simulink model "TosAeb"  (R2021a)
settings: Solver=FixedStepDiscrete  StartTime=0.0  StopTime=inf  FixedStep=0.01  RelTol=1e-3
undefined symbols (not defined in the file or in its folder): ACCModSts, AccSts1, AEB_ObjectMovingOrientation, AEB_ObjectMovingState, CN_BTCActive, CN_CurrentGearR, CN_CurrentGearR_DHT, CN_PTCActive, CN_VDCActive, CN_VSC_EspOff, CN_VSC_FCW_AEB_Error, CN_VSC_FCW_AEB_NoDispay, CN_VSC_FCW_AEB_PerfmDgdtn, CN_VSC_FCW_AEB_TempUnAvlbl, CN_VSC_VehStandstill, CNaccelAvoidMax, CNaccelAvoidMin, CNbrakePedalPress, CNcomfortStrLeftAccelMax, CNcomfortStrLeftAccelMin, CNcomfortStrRightAccelMax, CNcomfortStrRightAccelMin, CNDrvDoorOpen, CNDrvModeEco, CNDrvModeNorm, CNDrvModeOther, CNDrvModeSport, CNDrvSBRreminder, CNNull, CNobjInvalidID, CNPassDoorOpen, CNPassSBRreminder, CNPEBSAccelNegInf, CNPEBSAccelNull, CNPEBSTimeInf, CNPEBSTimeNull, CNSTOPMAX, CNSTOPNULL, CNsubjWidth, CNSwtOFF, CNSwtON, CNTTCMAX, CNTTCNULL, CNVehConfigAEBCycNone, CNVehConfigAEBNone, CNVehConfigAEBPedNone, CNVehConfigFCWNone, CNVehConfigJANone, CollisionSeverity, FALSE, FCWSnvty, JACycObjCalcInfoDefaultParams, JAPedObjCalcInfoDefaultParams, JAVehObjCalcInfoDefaultParams, latCycObjCalcInfoDefaultParams, latPedObjCalcInfoDefaultParams, longObjCalcInfoDefaultParams, ModSts, movingStatus, ObjectType ... (+1112)
libraries: simulink x3031, AEBSFunctionLib x50, Ta_Library x33, AEBSStateMachineLib x23, Mylibrary x12, simulink_extras x7, ObjSelect_lib x3, dspstat3 x2
(part 69 of 102)

== system TosAeb/FcwAebJa_Step_sys/ALG_AEBSystem/ALG_AEBController/AEB_JaObjCalcApp/JAVehTgtInfoCalc/AEBJAVehObjCalc/JAVehObjInfoCalc/ObjCrossFlgCal/CollisionProbCalc/Subsystem/ObjPreReferPointCalc ==
  dx : Inport  OutDataTypeStr=single
  vxAbsObj : Inport  Port=2  OutDataTypeStr=single
  tPredTime : Inport  Port=3  OutDataTypeStr=single
  axAbsObj : Inport  Port=4  OutDataTypeStr=single
  vy : Inport  Port=5  OutDataTypeStr=single
  subjLatAccel : Inport  Port=6
  ay : Inport  Port=7  OutDataTypeStr=single
  dy : Inport  Port=8  OutDataTypeStr=single
  ObjectYawAngle : Inport  Port=9  OutDataTypeStr=single
  Add : Sum  Inputs=+++  OutDataTypeStr=single
  Add1 : Sum  Inputs=+++  OutDataTypeStr=single
  Add2 : Sum  OutDataTypeStr=single
  Degrees to Radians : simulink_extras/Transformations/Degrees to Radians
  Gain : Gain  Gain=0.5  OutDataTypeStr=single
  Gain1 : Gain  Gain=0.5  OutDataTypeStr=single
  Math Function : Math  Operator=square  SignedPower=on  OutDataTypeStr=single  SaturateOnIntegerOverflow=on
  Math Function1 : Math  Operator=square  SignedPower=on  OutDataTypeStr=single  SaturateOnIntegerOverflow=on
  Product{,1..3} (4 alike) : Product  OutDataTypeStr=single
  dxPreRefPointObj : Outport
  dyPreRefPointObj : Outport  Port=2
  YawAnglePreObj : Outport  Port=3
  wires:
  vxAbsObj/1 -> Product/1
  tPredTime/1 -> Product/2, Math Function/1, Math Function1/1, Product2/2
  Product/1 -> Add/2
  Math Function/1 -> Product1/1
  axAbsObj/1 -> Product1/2
  Product1/1 -> Gain/1
  Gain/1 -> Add/3
  Add/1 -> dxPreRefPointObj/1
  dx/1 -> Add/1
  vy/1 -> Product2/1
  Math Function1/1 -> Product3/1
  Product3/1 -> Gain1/1
  Product2/1 -> Add1/1
  Gain1/1 -> Add1/2
  dy/1 -> Add1/3
  Add1/1 -> dyPreRefPointObj/1
  subjLatAccel/1 -> Add2/1
  ay/1 -> Add2/2
  Add2/1 -> Product3/2
  Degrees to Radians/1 -> YawAnglePreObj/1
  ObjectYawAngle/1 -> Degrees to Radians/1

== system TosAeb/FcwAebJa_Step_sys/ALG_AEBSystem/ALG_AEBController/AEB_JaObjCalcApp/JAVehTgtInfoCalc/AEBJAVehObjCalc/JAVehObjInfoCalc/ObjCrossFlgCal/CollisionProbCalc/Subsystem/PreCrossFlgCalcFlow/VectorCrossAlgorithm ==
  x1 : Inport  OutDataTypeStr=single
  y1 : Inport  Port=2  OutDataTypeStr=single
  x2 : Inport  Port=3  OutDataTypeStr=single
  y2 : Inport  Port=4  OutDataTypeStr=single
  m1 : Inport  Port=5  OutDataTypeStr=single
  n1 : Inport  Port=6  OutDataTypeStr=single
  m2 : Inport  Port=7  OutDataTypeStr=single
  n2 : Inport  Port=8  OutDataTypeStr=single
  f : TriggerPort  TriggerType=function-call  VariantControl=Variant
      settings: CopyFcn=Stateflow.SLINSF.SimulinkMan.removeBlockPreDeleteFcn  PreDeleteFcn=Stateflow.SLINSF.SimulinkMan.unsafePredeleteFcn
  Abs : Abs
  Bus Creator : BusCreator  Inputs=8
  Bus Selector : BusSelector  OutputSignals=x1,x2,n2,n1,y2,y1,m2,m1
  Compare To Constant : simulink/Logic and Bit Operations/Compare To Constant  relop=<  const=1e-6  OutDataTypeStr=boolean
  If : If  IfExpression=u1
  If Action Subsystem : Subsystem  TreatAsAtomicUnit=on
  If Action Subsystem1 : Subsystem  TreatAsAtomicUnit=on
  Merge : Merge
  VectorMulit : Subsystem
  preCrossFlg : Outport
  wires:
  x1/1 -> Bus Creator/1  "x1"
  y1/1 -> Bus Creator/2  "y1"
  x2/1 -> Bus Creator/3  "x2"
  y2/1 -> Bus Creator/4  "y2"
  m1/1 -> Bus Creator/5  "m1"
  n1/1 -> Bus Creator/6  "n1"
  m2/1 -> Bus Creator/7  "m2"
  n2/1 -> Bus Creator/8  "n2"
  Bus Creator/1 -> If Action Subsystem1/2(Coordinations), Bus Selector/1
  VectorMulit/1(vectorMulit) -> If Action Subsystem1/1(vectorMulit), Abs/1
  Abs/1 -> Compare To Constant/1
  Compare To Constant/1 -> If/1
  If/1 -> If Action Subsystem/Action
  If/2 -> If Action Subsystem1/Action
  If Action Subsystem/1(CrossFlg) -> Merge/1
  If Action Subsystem1/1(CrossFlg) -> Merge/2
  Merge/1 -> preCrossFlg/1
  Bus Selector/1 -> VectorMulit/1(x1)  "<x1>"
  Bus Selector/2 -> VectorMulit/2(x2)  "<x2>"
  Bus Selector/3 -> VectorMulit/3(n2)  "<n2>"
  Bus Selector/4 -> VectorMulit/4(n1)  "<n1>"
  Bus Selector/5 -> VectorMulit/5(y2)  "<y2>"
  Bus Selector/6 -> VectorMulit/6(y1)  "<y1>"
  Bus Selector/7 -> VectorMulit/7(m2)  "<m2>"
  Bus Selector/8 -> VectorMulit/8(m1)  "<m1>"

== system TosAeb/FcwAebJa_Step_sys/ALG_AEBSystem/ALG_AEBController/AEB_JaObjCalcApp/JAVehTgtInfoCalc/AEBJAVehObjCalc/JAVehObjInfoCalc/ObjCrossFlgCal/CollisionProbCalc/Subsystem/PreRefPointEgoCalc ==
  subjVel : Inport
  tPredTime : Inport  Port=2  OutDataTypeStr=single
  subjLongAccel : Inport  Port=3
  subjLatAccel : Inport  Port=4
  subjYawRate : Inport  Port=5
  Add : Sum  OutDataTypeStr=single
  Gain : Gain  Gain=0.5  OutDataTypeStr=single
  Gain1 : Gain  Gain=0.5  OutDataTypeStr=single
  Math Function : Math  Operator=square  SignedPower=on  OutDataTypeStr=single  SaturateOnIntegerOverflow=on
  Product{,1..3} (4 alike) : Product  OutDataTypeStr=single
  " dxPreRefPoint" : Outport
  " dyPreRefPoint" : Outport  Port=2
  YawAnglePre : Outport  Port=3
  wires:
  Gain1/1 -> " dyPreRefPoint"/1
  Gain/1 -> Add/2
  Product3/1 -> YawAnglePre/1
  subjYawRate/1 -> Product3/2
  Product2/1 -> Gain1/1
  subjLatAccel/1 -> Product2/2
  Add/1 -> " dxPreRefPoint"/1
  subjLongAccel/1 -> Product1/2
  Math Function/1 -> Product1/1, Product2/1
  tPredTime/1 -> Product3/1, Math Function/1, Product/2
  subjVel/1 -> Product/1
  Product/1 -> Add/1
  Product1/1 -> Gain/1

== system TosAeb/FcwAebJa_Step_sys/ALG_AEBSystem/ALG_AEBController/AEB_JaObjCalcApp/JAVehTgtInfoCalc/AEBJAVehObjCalc/JAVehObjInfoCalc/ObjCrossFlgCal/CollisionProbCalc/Subsystem/SubjBoundingBox ==
  dxPreRefPoint : Inport  OutDataTypeStr=single
  dyPreRefPoint : Inport  Port=2  OutDataTypeStr=single
  sincosCoef : Inport  Port=3
  Abs : Abs
  Bus Creator : BusCreator  Inputs=6
  Constant : Constant  Value=P_Global_DxvFrontBumper_m  OutDataTypeStr=single
  Constant1 : Constant  Value=P_Global_DxvRearBumper_m  OutDataTypeStr=single
  Constant2 : Constant  Value=P_Global_VehicleWidthMin_m  OutDataTypeStr=single
  Mux : Mux
  Mux1 : Mux
  SubjLLCoordinate : Subsystem
  SubjLUCoordinate : Subsystem
  SubjRLCoordinate : Subsystem
  SubjRUCoordinate : Subsystem
  subjDxPre : Outport
  subjDyPre : Outport  Port=2
  wires:
  Constant2/1 -> Bus Creator/6  "subjWidth"
  Abs/1 -> Bus Creator/5  "dxRearBumperToRearAxis"
  Constant/1 -> Bus Creator/4  "dxFrontBumperToRearAxis"
  sincosCoef/1 -> Bus Creator/3  "sincosCoef"
  dyPreRefPoint/1 -> Bus Creator/2  "dyPreRefPoint"
  dxPreRefPoint/1 -> Bus Creator/1  "dxPreRefPoint"
  Bus Creator/1 -> SubjRLCoordinate/1(inputSignals), SubjRUCoordinate/1(inputSignals), SubjLLCoordinate/1(inputSignals), SubjLUCoordinate/1(inputSignals)
  SubjLUCoordinate/1(dxPreLU) -> [dxPreLU]  "dxPreLU"
  SubjLUCoordinate/2(dyPreLU) -> [dyPreLU]  "dyPreLU"
  SubjLLCoordinate/1(dxPreLL) -> [dxPreLL]  "dxPreLL"
  SubjLLCoordinate/2(dyPreLL) -> [dyPreLL]  "dyPreLL"
  SubjRUCoordinate/2(dyPreRU) -> [dyPreRU]  "dyPreRU"
  SubjRLCoordinate/1(dxPreRL) -> [dxPreRL]  "dxPreRL"
  SubjRLCoordinate/2(dyPreRL) -> [dyPreRL]  "dyPreRL"
  Mux/1 -> subjDxPre/1
  Mux1/1 -> subjDyPre/1
  [dxPreRU] -> Mux/1
  [dxPreLU] -> Mux/2
  [dxPreLL] -> Mux/3
  [dxPreRL] -> Mux/4
  [dyPreRU] -> Mux1/1
  [dyPreLU] -> Mux1/2
  [dyPreRL] -> Mux1/4
  SubjRUCoordinate/1(dxPreRU) -> [dxPreRU]  "dyPreLU"
  [dyPreLL] -> Mux1/3
  Constant1/1 -> Abs/1

== system TosAeb/FcwAebJa_Step_sys/ALG_AEBSystem/ALG_AEBController/AEB_JaObjCalcApp/JAVehTgtInfoCalc/AEBJAVehObjCalc/JAVehObjInfoCalc/ObjCrossFlgCal/CollisionProbCalc/Subsystem/sincosCoefCalc ==
  angle : Inport  OutDataTypeStr=single
  Bus Creator : BusCreator  Inputs=2
  Sincos : Trigonometry  Operator=sincos
  sincosCoef : Outport
  wires:
  Sincos/1 -> Bus Creator/1  "sinCoef"
  angle/1 -> Sincos/1
  Sincos/2 -> Bus Creator/2  "cosCoef"
  Bus Creator/1 -> sincosCoef/1  "sincosCoef"

== system TosAeb/FcwAebJa_Step_sys/ALG_AEBSystem/ALG_AEBController/AEB_JaObjCalcApp/JAVehTgtInfoCalc/AEBJAVehObjCalc/JAVehObjInfoCalc/ObjCrossFlgCal/CollisionProbCalc/Subsystem/sincosCoefCalc1 ==
  angle : Inport  OutDataTypeStr=single
  Bus Creator : BusCreator  Inputs=2
  Sincos : Trigonometry  Operator=sincos
  sincosCoef : Outport
  wires:
  Bus Creator/1 -> sincosCoef/1  "sincosCoef"
  Sincos/2 -> Bus Creator/2  "cosCoef"
  angle/1 -> Sincos/1
  Sincos/1 -> Bus Creator/1  "sinCoef"

== system TosAeb/FcwAebJa_Step_sys/ALG_AEBSystem/ALG_AEBController/AEB_JaObjCalcApp/JAVehTgtInfoCalc/JAVehCollisonAssessInfoCalc/JaVehCollisonAssessInfoCalc/VehDistBrakeFullCalc/DistPhase3Calc/tPhase3Calc/tEgoStopCalc ==
  subjVel : Inport  OutDataTypeStr=single
  subjLongAccelFilt : Inport  Port=2  OutDataTypeStr=single
  tJerkWork : Inport  Port=3  OutDataTypeStr=single
  Kjerk : Inport  Port=4  OutDataTypeStr=single
  accelMax : Inport  Port=5  OutDataTypeStr=single
  Add : Sum  Inputs=3  OutDataTypeStr=single
  Constant : Constant  Value=0.5  OutDataTypeStr=single
  Divide : Product  Inputs=*/  OutDataTypeStr=single
  Gain : Gain  Gain=-1  OutDataTypeStr=single
  Product : Product  Inputs=4  OutDataTypeStr=single
  Product1 : Product  OutDataTypeStr=single
  tEgoStop : Outport
  wires:
  Divide/1 -> Gain/1
  Product/1 -> Add/3
  Product1/1 -> Add/2
  tJerkWork/1 -> Product/2, Product/1, Product1/2
  Add/1 -> Divide/1
  Gain/1 -> tEgoStop/1
  accelMax/1 -> Divide/2
  Kjerk/1 -> Product/3
  subjLongAccelFilt/1 -> Product1/1
  Constant/1 -> Product/4
  subjVel/1 -> Add/1

== system TosAeb/FcwAebJa_Step_sys/ALG_AEBSystem/ALG_AEBController/AEB_JaObjCalcApp/JAVehTgtInfoCalc/JAVehCollisonAssessInfoCalc/JaVehCollisonAssessInfoCalc/VehDistBrakeFullCalc/DistPhase3Calc/tPhase3Calc/tPhase3Calc ==
  tVelDelta : Inport  OutDataTypeStr=single
  tEgoStop : Inport  Port=2  OutDataTypeStr=single
  MinMax : MinMax  Inputs=2  OutDataTypeStr=single
  tPhase3 : Outport
  wires:
  tVelDelta/1 -> MinMax/1
  tEgoStop/1 -> MinMax/2
  MinMax/1 -> tPhase3/1

== system TosAeb/FcwAebJa_Step_sys/ALG_AEBSystem/ALG_AEBController/AEB_JaObjCalcApp/JAVehTgtInfoCalc/JAVehCollisonAssessInfoCalc/JaVehCollisonAssessInfoCalc/VehDistBrakeFullCalc/DistPhase3Calc/tPhase3Calc/tVelDeltaCalc ==
  velDelta : Inport  OutDataTypeStr=single
  subjLongAccelFilt : Inport  Port=2  OutDataTypeStr=single
  tJerkWork : Inport  Port=3  OutDataTypeStr=single
  Kjerk : Inport  Port=4  OutDataTypeStr=single
  accelMax : Inport  Port=5  OutDataTypeStr=single
  Add : Sum  Inputs=+--  OutDataTypeStr=single
  Constant : Constant  Value=0.5  OutDataTypeStr=single
  Divide : Product  Inputs=*/  OutDataTypeStr=single
  Product : Product  Inputs=4  OutDataTypeStr=single
  Product1 : Product  OutDataTypeStr=single
  tVelDelta : Outport
  wires:
  Divide/1 -> tVelDelta/1
  accelMax/1 -> Divide/2
  Kjerk/1 -> Product/3
  velDelta/1 -> Add/1
  Add/1 -> Divide/1
  Product1/1 -> Add/2
  Constant/1 -> Product/4
  tJerkWork/1 -> Product/2, Product/1, Product1/2
  Product/1 -> Add/3
  subjLongAccelFilt/1 -> Product1/1

== system TosAeb/FcwAebJa_Step_sys/ALG_AEBSystem/ALG_AEBController/AEB_JaObjCalcApp/JaCycTgtInfoCalc/JaCycCollisonAssessInfo/JaCycCollisonAssessInfoCalc/PedDistBrakeFullCalc/DistPhase3Calc/tPhase3Calc/tEgoStopCalc ==
  subjVel : Inport  OutDataTypeStr=single
  subjLongAccelFilt : Inport  Port=2  OutDataTypeStr=single
  tJerkWork : Inport  Port=3  OutDataTypeStr=single
  Kjerk : Inport  Port=4  OutDataTypeStr=single
  accelMax : Inport  Port=5  OutDataTypeStr=single
  Add : Sum  Inputs=3  OutDataTypeStr=single
  Constant : Constant  Value=0.5  OutDataTypeStr=single
  Divide : Product  Inputs=*/  OutDataTypeStr=single
  Gain : Gain  Gain=-1  OutDataTypeStr=single
  Product : Product  Inputs=4  OutDataTypeStr=single
  Product1 : Product  OutDataTypeStr=single
  tEgoStop : Outport
  wires:
  Divide/1 -> Gain/1
  Product/1 -> Add/3
  Product1/1 -> Add/2
  tJerkWork/1 -> Product/2, Product/1, Product1/2
  Add/1 -> Divide/1
  Gain/1 -> tEgoStop/1
  accelMax/1 -> Divide/2
  Kjerk/1 -> Product/3
  subjLongAccelFilt/1 -> Product1/1
  Constant/1 -> Product/4
  subjVel/1 -> Add/1

== system TosAeb/FcwAebJa_Step_sys/ALG_AEBSystem/ALG_AEBController/AEB_JaObjCalcApp/JaCycTgtInfoCalc/JaCycCollisonAssessInfo/JaCycCollisonAssessInfoCalc/PedDistBrakeFullCalc/DistPhase3Calc/tPhase3Calc/tPhase3Calc ==
  tVelDelta : Inport  OutDataTypeStr=single
  tEgoStop : Inport  Port=2  OutDataTypeStr=single
  MinMax : MinMax  Inputs=2  OutDataTypeStr=single
  tPhase3 : Outport
  wires:
  tVelDelta/1 -> MinMax/1
  tEgoStop/1 -> MinMax/2
  MinMax/1 -> tPhase3/1

== system TosAeb/FcwAebJa_Step_sys/ALG_AEBSystem/ALG_AEBController/AEB_JaObjCalcApp/JaCycTgtInfoCalc/JaCycCollisonAssessInfo/JaCycCollisonAssessInfoCalc/PedDistBrakeFullCalc/DistPhase3Calc/tPhase3Calc/tVelDeltaCalc ==
  velDelta : Inport  OutDataTypeStr=single
  subjLongAccelFilt : Inport  Port=2  OutDataTypeStr=single
  tJerkWork : Inport  Port=3  OutDataTypeStr=single
  Kjerk : Inport  Port=4  OutDataTypeStr=single
  accelMax : Inport  Port=5  OutDataTypeStr=single
  Add : Sum  Inputs=+--  OutDataTypeStr=single
  Constant : Constant  Value=0.5  OutDataTypeStr=single
  Divide : Product  Inputs=*/  OutDataTypeStr=single
  Product : Product  Inputs=4  OutDataTypeStr=single
  Product1 : Product  OutDataTypeStr=single
  tVelDelta : Outport
  wires:
  Divide/1 -> tVelDelta/1
  accelMax/1 -> Divide/2
  Kjerk/1 -> Product/3
  velDelta/1 -> Add/1
  Add/1 -> Divide/1
  Product1/1 -> Add/2
  Constant/1 -> Product/4
  tJerkWork/1 -> Product/2, Product/1, Product1/2
  Product/1 -> Add/3
  subjLongAccelFilt/1 -> Product1/1

== system TosAeb/FcwAebJa_Step_sys/ALG_AEBSystem/ALG_AEBController/AEB_JaObjCalcApp/JaCycTgtInfoCalc/JaCycObjCalcInfoCalc/JaCycObjInfoCalc/ObjCrossFlgCal/CollisionProbCalc/Subsystem/ObjBoundingBox ==
  ObjLengthCorr : Inport  OutDataTypeStr=single
  ObjWidthCorr : Inport  Port=2  OutDataTypeStr=single
  dxPreRefPointObj : Inport  Port=3  OutDataTypeStr=single
  dyPreRefPointObj : Inport  Port=4  OutDataTypeStr=single
  sincosCoef : Inport  Port=5
  Bus Creator : BusCreator  Inputs=5
  Mux : Mux
  Mux1 : Mux
  ObjLLCoordinate : Subsystem
  ObjLUCoordinate : Subsystem
  ObjRLCoordinate : Subsystem
  ObjRUCoordinate : Subsystem
  objDxPre : Outport
  objDyPre : Outport  Port=2
  wires:
  ObjLengthCorr/1 -> Bus Creator/1  "ObjLengthCorr"
  ObjWidthCorr/1 -> Bus Creator/2  "ObjWidthCorr"
  dxPreRefPointObj/1 -> Bus Creator/3  "dxPreRefPointObj"
  dyPreRefPointObj/1 -> Bus Creator/4  "dyPreRefPointObj"
  sincosCoef/1 -> Bus Creator/5  "sincosCoef"
  Bus Creator/1 -> ObjRLCoordinate/1(inputSignals), ObjRUCoordinate/1(inputSignals), ObjLLCoordinate/1(inputSignals), ObjLUCoordinate/1(inputSignals)
  ObjLUCoordinate/1(dxPreLUObj) -> [dxPreLUObj]  "dxPreLUObj"
  ObjLUCoordinate/2(dyPreLUobj) -> [dyPreLUObj]  "dyPreLUObj"
  ObjLLCoordinate/1(dxPre_LLObj) -> [dxPreLLObj]  "dxPreLLObj"
  ObjLLCoordinate/2(dyPreLLObj) -> [dyPreLLObj]  "dyPreLLObj"
  ObjRUCoordinate/1(dxPreRUObj) -> [dxPreRUObj]  "dxPreRUObj"
  ObjRUCoordinate/2(dyPreRUObj) -> [dyPreRUObj]  "dyPreRUObj"
  ObjRLCoordinate/1(dxPreRLObj) -> [dxPreRLObj]  "dxPreRLObj"
  ObjRLCoordinate/2(dyPreRLObj) -> [dyPreRLObj]  "dyPreRLObj"
  Mux/1 -> objDxPre/1
  Mux1/1 -> objDyPre/1
  [dxPreRUObj] -> Mux/1  "dxPreRUObj"
  [dxPreLUObj] -> Mux/2
  [dxPreLLObj] -> Mux/3
  [dxPreRLObj] -> Mux/4
  [dyPreRUObj] -> Mux1/1
  [dyPreLUObj] -> Mux1/2
  [dyPreLLObj] -> Mux1/3
  [dyPreRLObj] -> Mux1/4

== system TosAeb/FcwAebJa_Step_sys/ALG_AEBSystem/ALG_AEBController/AEB_JaObjCalcApp/JaCycTgtInfoCalc/JaCycObjCalcInfoCalc/JaCycObjInfoCalc/ObjCrossFlgCal/CollisionProbCalc/Subsystem/ObjLenthWidthCalc ==
  ObjectYawAngleCorr : Inport  OutDataTypeStr=single
  ObjectTypeCameraConfirmed : Inport  Port=2
  ObjectLength : Inport  Port=3  OutDataTypeStr=single
  ObjectWidth : Inport  Port=4  OutDataTypeStr=single
  ObjectMovingOrientation : Inport  Port=5
  ObjLengthWidthCalc : Stateflow chart  Commented=on  TreatAsAtomicUnit=on
  ObjLengthWidthCalc1 : Stateflow chart  TreatAsAtomicUnit=on
  P_LatPedCA_CollisionRiskProbLengthThres : Constant  Value=P_AEB_JaCycCA_PreCollisionCycLengthThres
  P_LatPedCA_CollisionRiskProbWidthThres : Constant  Value=P_AEB_JaCycCA_PreCollisionCycWidthThres
  Radians to Degrees : simulink_extras/Transformations/Radians to Degrees
  ObjLengthCorr : Outport
  ObjWidthCorr : Outport  Port=2
  wires:
  P_LatPedCA_CollisionRiskProbWidthThres/1 -> ObjLengthWidthCalc1/7(P_LatPedCA_CollisionRiskProbWidthThres)
  ObjectTypeCameraConfirmed/1 -> ObjLengthWidthCalc1/2(ObjectTypeCameraConfirmed)
  P_LatPedCA_CollisionRiskProbLengthThres/1 -> ObjLengthWidthCalc1/6(P_LatPedCA_CollisionRiskProbLengthThres)
  ObjLengthWidthCalc1/1(ObjectLength) -> ObjLengthCorr/1
  ObjLengthWidthCalc1/2(ObjectWidth) -> ObjWidthCorr/1
  ObjectMovingOrientation/1 -> ObjLengthWidthCalc1/5(ObjectMovingOrientation)
  ObjectYawAngleCorr/1 -> Radians to Degrees/1
  ObjectLength/1 -> ObjLengthWidthCalc1/3(ObjectLengthIn)
  ObjectWidth/1 -> ObjLengthWidthCalc1/4(ObjectWidthIn)
  Radians to Degrees/1 -> ObjLengthWidthCalc1/1(ObjectYawAngle)

== system TosAeb/FcwAebJa_Step_sys/ALG_AEBSystem/ALG_AEBController/AEB_JaObjCalcApp/JaCycTgtInfoCalc/JaCycObjCalcInfoCalc/JaCycObjInfoCalc/ObjCrossFlgCal/CollisionProbCalc/Subsystem/ObjPreReferPointCalc ==
  dx : Inport  OutDataTypeStr=single
  vxAbsObj : Inport  Port=2  OutDataTypeStr=single
  tPredTime : Inport  Port=3  OutDataTypeStr=single
  vyRelCorr : Inport  Port=4  OutDataTypeStr=single
  dy : Inport  Port=5  OutDataTypeStr=single
  ObjectYawObjectYawAngleCorrAngle : Inport  Port=6  OutDataTypeStr=single
  Add : Sum  OutDataTypeStr=single
  Add1 : Sum  OutDataTypeStr=single
  Product : Product  OutDataTypeStr=single
  Product2 : Product  OutDataTypeStr=single
  dxPreRefPointObj : Outport
  dyPreRefPointObj : Outport  Port=2
  YawAnglePreObj : Outport  Port=3
  wires:
  vxAbsObj/1 -> Product/1
  tPredTime/1 -> Product/2, Product2/2
  Product/1 -> Add/2
  Add/1 -> dxPreRefPointObj/1
  dx/1 -> Add/1
  vyRelCorr/1 -> Product2/1
  Product2/1 -> Add1/1
  dy/1 -> Add1/2
  Add1/1 -> dyPreRefPointObj/1
  ObjectYawObjectYawAngleCorrAngle/1 -> YawAnglePreObj/1

== system TosAeb/FcwAebJa_Step_sys/ALG_AEBSystem/ALG_AEBController/AEB_JaObjCalcApp/JaCycTgtInfoCalc/JaCycObjCalcInfoCalc/JaCycObjInfoCalc/ObjCrossFlgCal/CollisionProbCalc/Subsystem/PreCrossFlgCalcFlow/VectorCrossAlgorithm ==
  x1 : Inport  OutDataTypeStr=single
  y1 : Inport  Port=2  OutDataTypeStr=single
  x2 : Inport  Port=3  OutDataTypeStr=single
  y2 : Inport  Port=4  OutDataTypeStr=single
  m1 : Inport  Port=5  OutDataTypeStr=single
  n1 : Inport  Port=6  OutDataTypeStr=single
  m2 : Inport  Port=7  OutDataTypeStr=single
  n2 : Inport  Port=8  OutDataTypeStr=single
  f : TriggerPort  TriggerType=function-call  VariantControl=Variant
      settings: CopyFcn=Stateflow.SLINSF.SimulinkMan.removeBlockPreDeleteFcn  PreDeleteFcn=Stateflow.SLINSF.SimulinkMan.unsafePredeleteFcn
  Abs : Abs
  Bus Creator : BusCreator  Inputs=8
  Bus Selector : BusSelector  OutputSignals=x1,x2,n2,n1,y2,y1,m2,m1
  Compare To Constant : simulink/Logic and Bit Operations/Compare To Constant  relop=<  const=1e-6  OutDataTypeStr=boolean
  If : If  IfExpression=u1
  If Action Subsystem : Subsystem  TreatAsAtomicUnit=on
  If Action Subsystem1 : Subsystem  TreatAsAtomicUnit=on
  Merge : Merge
  VectorMulit : Subsystem
  preCrossFlg : Outport
  wires:
  x1/1 -> Bus Creator/1  "x1"
  y1/1 -> Bus Creator/2  "y1"
  x2/1 -> Bus Creator/3  "x2"
  y2/1 -> Bus Creator/4  "y2"
  m1/1 -> Bus Creator/5  "m1"
  n1/1 -> Bus Creator/6  "n1"
  m2/1 -> Bus Creator/7  "m2"
  n2/1 -> Bus Creator/8  "n2"
  Bus Creator/1 -> If Action Subsystem1/2(Coordinations), Bus Selector/1
  VectorMulit/1(vectorMulit) -> If Action Subsystem1/1(vectorMulit), Abs/1
  Abs/1 -> Compare To Constant/1
  Compare To Constant/1 -> If/1
  If/1 -> If Action Subsystem/Action
  If/2 -> If Action Subsystem1/Action
  If Action Subsystem/1(CrossFlg) -> Merge/1
  If Action Subsystem1/1(CrossFlg) -> Merge/2
  Merge/1 -> preCrossFlg/1
  Bus Selector/1 -> VectorMulit/1(x1)  "<x1>"
  Bus Selector/2 -> VectorMulit/2(x2)  "<x2>"
  Bus Selector/3 -> VectorMulit/3(n2)  "<n2>"
  Bus Selector/4 -> VectorMulit/4(n1)  "<n1>"
  Bus Selector/5 -> VectorMulit/5(y2)  "<y2>"
  Bus Selector/6 -> VectorMulit/6(y1)  "<y1>"
  Bus Selector/7 -> VectorMulit/7(m2)  "<m2>"
  Bus Selector/8 -> VectorMulit/8(m1)  "<m1>"

== system TosAeb/FcwAebJa_Step_sys/ALG_AEBSystem/ALG_AEBController/AEB_JaObjCalcApp/JaCycTgtInfoCalc/JaCycObjCalcInfoCalc/JaCycObjInfoCalc/ObjCrossFlgCal/CollisionProbCalc/Subsystem/PreRefPointEgoCalc ==
  subjVel : Inport  OutDataTypeStr=single
  tPredTime : Inport  Port=2  OutDataTypeStr=single
  subjLongAccel : Inport  Port=3  OutDataTypeStr=single
  subjLatAccel : Inport  Port=4  OutDataTypeStr=single
  subjYawRate : Inport  Port=5  OutDataTypeStr=single
  Add : Sum  OutDataTypeStr=single
  Gain : Gain  Gain=0.5  OutDataTypeStr=single
  Gain1 : Gain  Gain=0.5  OutDataTypeStr=single
  Math Function : Math  Operator=square  SignedPower=on  OutDataTypeStr=single  SaturateOnIntegerOverflow=on
  Product{,1..3} (4 alike) : Product  OutDataTypeStr=single
  " dxPreRefPoint" : Outport
  " dyPreRefPoint" : Outport  Port=2
  YawAnglePre : Outport  Port=3
  wires:
  Gain1/1 -> " dyPreRefPoint"/1
  Gain/1 -> Add/2
  Product3/1 -> YawAnglePre/1
  subjYawRate/1 -> Product3/2
  Product2/1 -> Gain1/1
  subjLatAccel/1 -> Product2/2
  Add/1 -> " dxPreRefPoint"/1
  subjLongAccel/1 -> Product1/2
  Math Function/1 -> Product1/1, Product2/1
  tPredTime/1 -> Product3/1, Math Function/1, Product/2
  subjVel/1 -> Product/1
  Product/1 -> Add/1
  Product1/1 -> Gain/1

== system TosAeb/FcwAebJa_Step_sys/ALG_AEBSystem/ALG_AEBController/AEB_JaObjCalcApp/JaCycTgtInfoCalc/JaCycObjCalcInfoCalc/JaCycObjInfoCalc/ObjCrossFlgCal/CollisionProbCalc/Subsystem/SubjBoundingBox ==
  dxPreRefPoint : Inport  OutDataTypeStr=single
  dyPreRefPoint : Inport  Port=2  OutDataTypeStr=single
  sincosCoef : Inport  Port=3
  Abs : Abs
  Bus Creator : BusCreator  Inputs=6
  Constant : Constant  Value=P_Global_DxvFrontBumper_m  OutDataTypeStr=single
  Constant1 : Constant  Value=P_Global_DxvRearBumper_m  OutDataTypeStr=single
  Constant2 : Constant  Value=P_Global_VehicleWidthMin_m  OutDataTypeStr=single
  Mux : Mux
  Mux1 : Mux
  SubjLLCoordinate : Subsystem
  SubjLUCoordinate : Subsystem
  SubjRLCoordinate : Subsystem
  SubjRUCoordinate : Subsystem
  subjDxPre : Outport
  subjDyPre : Outport  Port=2
  wires:
  Constant2/1 -> Bus Creator/6  "subjWidth"
  Abs/1 -> Bus Creator/5  "dxRearBumperToRearAxis"
  Constant/1 -> Bus Creator/4  "dxFrontBumperToRearAxis"
  sincosCoef/1 -> Bus Creator/3  "sincosCoef"
  dyPreRefPoint/1 -> Bus Creator/2  "dyPreRefPoint"
  dxPreRefPoint/1 -> Bus Creator/1  "dxPreRefPoint"
  Bus Creator/1 -> SubjRLCoordinate/1(inputSignals), SubjRUCoordinate/1(inputSignals), SubjLLCoordinate/1(inputSignals), SubjLUCoordinate/1(inputSignals)
  SubjLUCoordinate/1(dxPreLU) -> [dxPreLU]  "dxPreLU"
  SubjLUCoordinate/2(dyPreLU) -> [dyPreLU]  "dyPreLU"
  SubjLLCoordinate/1(dxPreLL) -> [dxPreLL]  "dxPreLL"
  SubjLLCoordinate/2(dyPreLL) -> [dyPreLL]  "dyPreLL"
  SubjRUCoordinate/1(dxPreRU) -> [dxPreRU]  "dxPreRU"
  SubjRUCoordinate/2(dyPreRU) -> [dyPreRU]  "dyPreRU"
  SubjRLCoordinate/1(dxPreRL) -> [dxPreRL]  "dxPreRL"
  SubjRLCoordinate/2(dyPreRL) -> [dyPreRL]  "dyPreRL"
  Mux/1 -> subjDxPre/1
  Mux1/1 -> subjDyPre/1
  [dxPreRU] -> Mux/1
  [dxPreLU] -> Mux/2
  [dxPreLL] -> Mux/3
  [dxPreRL] -> Mux/4
  [dyPreRU] -> Mux1/1
  [dyPreLU] -> Mux1/2
  [dyPreLL] -> Mux1/3
  [dyPreRL] -> Mux1/4
  Constant1/1 -> Abs/1

== system TosAeb/FcwAebJa_Step_sys/ALG_AEBSystem/ALG_AEBController/AEB_JaObjCalcApp/JaCycTgtInfoCalc/JaCycObjCalcInfoCalc/JaCycObjInfoCalc/ObjCrossFlgCal/CollisionProbCalc/Subsystem/sincosCoefCalc ==
  angle : Inport  OutDataTypeStr=single
  Bus Creator : BusCreator  Inputs=2
  Sincos : Trigonometry  Operator=sincos
  sincosCoef : Outport
  wires:
  Sincos/1 -> Bus Creator/1  "sinCoef"
  angle/1 -> Sincos/1
  Sincos/2 -> Bus Creator/2  "cosCoef"
  Bus Creator/1 -> sincosCoef/1  "sincosCoef"

== system TosAeb/FcwAebJa_Step_sys/ALG_AEBSystem/ALG_AEBController/AEB_JaObjCalcApp/JaCycTgtInfoCalc/JaCycObjCalcInfoCalc/JaCycObjInfoCalc/ObjCrossFlgCal/CollisionProbCalc/Subsystem/sincosCoefCalc1 ==
  angle : Inport  OutDataTypeStr=single
  Bus Creator : BusCreator  Inputs=2
  Sincos : Trigonometry  Operator=sincos
  sincosCoef : Outport
  wires:
  Bus Creator/1 -> sincosCoef/1  "sincosCoef"
  Sincos/2 -> Bus Creator/2  "cosCoef"
  angle/1 -> Sincos/1
  Sincos/1 -> Bus Creator/1  "sinCoef"

== system TosAeb/FcwAebJa_Step_sys/ALG_AEBSystem/ALG_AEBController/AEB_LatObjInfoCalcApp/LatCycObjCalc/LatCycCollisonAssessInfoCalc/LatCycCollisonAssessInfoCalc/DistBrkCondCalc/CycDistBrakeFullCalc/DistPhase2Calc/distPhase2Calc ==
  tPhase2 : Inport  OutDataTypeStr=single
  subjAccelFilt : Inport  Port=2  OutDataTypeStr=single
  Kjerk : Inport  Port=3  OutDataTypeStr=single
  subjVel : Inport  Port=4
  Add : Sum  Inputs=3  OutDataTypeStr=single
  Constant : Constant  Value=1/6  OutDataTypeStr=single
  Constant1 : Constant  Value=0.5  OutDataTypeStr=single
  Product : Product  Inputs=5  OutDataTypeStr=single
  Product1 : Product  Inputs=4  OutDataTypeStr=single
  Product2 : Product  OutDataTypeStr=single
  distPhase2 : Outport
  wires:
  tPhase2/1 -> Product1/3, Product1/4, Product2/2, Product/1, Product/2, Product/3
  Kjerk/1 -> Product/4
  Constant/1 -> Product/5
  subjAccelFilt/1 -> Product1/2
  Constant1/1 -> Product1/1
  subjVel/1 -> Product2/1
  Product2/1 -> Add/2
  Product/1 -> Add/3
  Product1/1 -> Add/1
  Add/1 -> distPhase2/1

== system TosAeb/FcwAebJa_Step_sys/ALG_AEBSystem/ALG_AEBController/AEB_LatObjInfoCalcApp/LatCycObjCalc/LatCycCollisonAssessInfoCalc/LatCycCollisonAssessInfoCalc/DistBrkCondCalc/CycDistBrakeFullCalc/DistPhase2Calc/tPhase2Calc ==
  note: t jerk_amax = Max[0 ，（ a m ax - a ego ） /K jerk ] ；
  note: t jerk_0 = （ -a ego /K jer k ） + sqrt （（ a ego /K jer k ） ²- 2* （ v ego / K jer k ））
  subjVel : Inport
  Kjerk : Inport  Port=2  OutDataTypeStr=single
  subjLongAccelFilt : Inport  Port=3  OutDataTypeStr=single
  accelMax : Inport  Port=4  OutDataTypeStr=single
  Add : Sum  Inputs=+-  OutDataTypeStr=single
  Add1 : Sum  Inputs=-+  OutDataTypeStr=single
  Add2 : Sum  Inputs=+-  OutDataTypeStr=single
  Constant : Constant  Value=0  OutDataTypeStr=single
  Divide : Product  Inputs=*/  OutDataTypeStr=single
  Divide1 : Product  Inputs=*/  OutDataTypeStr=single
  Divide2 : Product  Inputs=*/  OutDataTypeStr=single
  Gain : Gain  Gain=2  OutDataTypeStr=single
  Math Function : Math  Operator=square  SignedPower=on  OutDataTypeStr=single  SaturateOnIntegerOverflow=on
  MinMax : MinMax  Function=max  Inputs=2  OutDataTypeStr=single
  MinMax1 : MinMax  Inputs=2  OutDataTypeStr=single
  Relational Operator : RelationalOperator  Operator=<=  OutDataTypeStr=boolean  RndMeth=Simplest
  Sqrt : Sqrt  OutDataTypeStr=single  SaturateOnIntegerOverflow=on
  egoStopFlg : Outport
  tJerkWork : Outport  Port=2
  tPhase2 : Outport  Port=3
  wires:
  Add2/1 -> Divide2/1
  MinMax/1 -> tJerkWork/1, Relational Operator/2, MinMax1/1  "tJerkWork"
  subjLongAccelFilt/1 -> Add2/2, Divide/1
  Sqrt/1 -> Add1/2
  Kjerk/1 -> Divide2/2, Divide/2, Divide1/2
  accelMax/1 -> Add2/1
  Divide2/1 -> MinMax/1
  Constant/1 -> MinMax/2
  Divide/1 -> Add1/1, Math Function/1
  MinMax1/1 -> tPhase2/1
  Divide1/1 -> Gain/1
  Add/1 -> Sqrt/1
  Gain/1 -> Add/2
  Add1/1 -> Relational Operator/1, MinMax1/2  "tEgoStop"
  Math Function/1 -> Add/1
  subjVel/1 -> Divide1/1
  Relational Operator/1 -> egoStopFlg/1

== system TosAeb/FcwAebJa_Step_sys/ALG_AEBSystem/ALG_AEBController/AEB_LatObjInfoCalcApp/LatCycObjCalc/LatCycCollisonAssessInfoCalc/LatCycCollisonAssessInfoCalc/DistBrkCondCalc/CycDistBrakeFullCalc/DistPhase3Calc/distPhase3Calc ==
  note: d3=（vego+ 0.5* aego*（t1 + t2）+ 0.5* Kjerk* t2²）*t3 + 0.5* amax* t3²；
  tPhase3 : Inport  OutDataTypeStr=single
  subjVel : Inport  Port=2
  subjLongAccelFilt : Inport  Port=3  OutDataTypeStr=single
  Kjerk : Inport  Port=4  OutDataTypeStr=single
  tPhase1 : Inport  Port=5  OutDataTypeStr=single
  tPhase2 : Inport  Port=6  OutDataTypeStr=single
  accelMax : Inport  Port=7  OutDataTypeStr=single
  Add : Sum  OutDataTypeStr=single
  Add1 : Sum  Inputs=+++  OutDataTypeStr=single
  Add2 : Sum  OutDataTypeStr=single
  Constant : Constant  Value=0.5  OutDataTypeStr=single
  Constant1 : Constant  Value=0.5  OutDataTypeStr=single
  Constant2 : Constant  Value=0.5  OutDataTypeStr=single
  Product : Product  Inputs=3  OutDataTypeStr=single
  Product1 : Product  Inputs=4  OutDataTypeStr=single
  Product2 : Product  OutDataTypeStr=single
  Product3 : Product  Inputs=4  OutDataTypeStr=single
  distPhase3 : Outport
  wires:
  tPhase1/1 -> Add/1
  tPhase2/1 -> Product1/2, Product1/1, Add/2
  subjLongAccelFilt/1 -> Product/2
  Add/1 -> Product/3
  Constant/1 -> Product/1
  Constant1/1 -> Product1/4
  Kjerk/1 -> Product1/3
  Product/1 -> Add1/2
  Product1/1 -> Add1/3
  subjVel/1 -> Add1/1
  Add1/1 -> Product2/2
  tPhase3/1 -> Product2/1, Product3/4, Product3/3
  Constant2/1 -> Product3/1
  accelMax/1 -> Product3/2
  Product3/1 -> Add2/1
  Product2/1 -> Add2/2
  Add2/1 -> distPhase3/1

== system TosAeb/FcwAebJa_Step_sys/ALG_AEBSystem/ALG_AEBController/AEB_LatObjInfoCalcApp/LatCycObjCalc/LatCycCollisonAssessInfoCalc/LatCycCollisonAssessInfoCalc/DistBrkCondCalc/CycDistBrakeFullCalc/DistPhase3Calc/tPhase3Calc ==
  subjVel : Inport
  subjLongAccelFilt : Inport  Port=2  OutDataTypeStr=single
  tJerkWork : Inport  Port=3  OutDataTypeStr=single
  Kjerk : Inport  Port=4  OutDataTypeStr=single
  accelMax : Inport  Port=5  OutDataTypeStr=single
  velDelta : Inport  Port=6  OutDataTypeStr=single
  Action Port : ActionPort  ActionPortLabel=else
  Bus Creator : BusCreator  Inputs=6
  Bus Selector : BusSelector  OutputSignals=velDelta,subjLongAccelFilt,tJerkWork,Kjerk,accelMax
  Bus Selector1 : BusSelector  OutputSignals=subjVel,subjLongAccelFilt,tJerkWork,Kjerk,accelMax
  tEgoStopCalc : Subsystem
  tPhase3Calc : Subsystem
  tVelDeltaCalc : Subsystem
  tPhase3 : Outport
  wires:
  tVelDeltaCalc/1(tVelDelta) -> tPhase3Calc/1(tVelDelta)
  tEgoStopCalc/1(tEgoStop) -> tPhase3Calc/2(tEgoStop)
  tPhase3Calc/1(tPhase3) -> tPhase3/1
  subjVel/1 -> Bus Creator/1  "subjVel"
  subjLongAccelFilt/1 -> Bus Creator/2  "subjLongAccelFilt"
  tJerkWork/1 -> Bus Creator/3  "tJerkWork"
  Kjerk/1 -> Bus Creator/4  "Kjerk"
  accelMax/1 -> Bus Creator/5  "accelMax"
  velDelta/1 -> Bus Creator/6  "velDelta"
  Bus Creator/1 -> Bus Selector1/1, Bus Selector/1
  Bus Selector/3 -> tVelDeltaCalc/3(tJerkWork)  "<tJerkWork>"
  Bus Selector/2 -> tVelDeltaCalc/2(subjLongAccelFilt)  "<subjLongAccelFilt>"
  Bus Selector/4 -> tVelDeltaCalc/4(Kjerk)  "<Kjerk>"
  Bus Selector/1 -> tVelDeltaCalc/1(velDelta)  "<velDelta>"
  Bus Selector/5 -> tVelDeltaCalc/5(accelMax)  "<accelMax>"
  Bus Selector1/1 -> tEgoStopCalc/1(subjVel)  "<subjVel>"
  Bus Selector1/2 -> tEgoStopCalc/2(subjLongAccelFilt)  "<subjLongAccelFilt>"
  Bus Selector1/3 -> tEgoStopCalc/3(tJerkWork)  "<tJerkWork>"
  Bus Selector1/4 -> tEgoStopCalc/4(Kjerk)  "<Kjerk>"
  Bus Selector1/5 -> tEgoStopCalc/5(accelMax)  "<accelMax>"

== system TosAeb/FcwAebJa_Step_sys/ALG_AEBSystem/ALG_AEBController/AEB_LatObjInfoCalcApp/LatCycObjCalc/LatCycCollisonAssessInfoCalc/LatCycCollisonAssessInfoCalc/DistBrkCondCalc/CycDistBrakeFullCalc/DistPhase3Calc/tPhase3Calc1 ==
  Action Port : ActionPort  ActionPortLabel=if(u1)
  Constant : Constant  Value=0  OutDataTypeStr=single
  tPhase3 : Outport
  wires:
  Constant/1 -> tPhase3/1

== system TosAeb/FcwAebJa_Step_sys/ALG_AEBSystem/ALG_AEBController/AEB_LatObjInfoCalcApp/LatCycObjCalc/LatCycCollisonAssessInfoCalc/LatCycCollisonAssessInfoCalc/DistBrkCondCalc/CycDistBrakePartCalc/DistPhase2Calc/distPhase2Calc ==
  tPhase2 : Inport  OutDataTypeStr=single
  subjAccelFilt : Inport  Port=2  OutDataTypeStr=single
  Kjerk : Inport  Port=3  OutDataTypeStr=single
  subjVel : Inport  Port=4
  Add : Sum  Inputs=3  OutDataTypeStr=single
  Constant : Constant  Value=1/6  OutDataTypeStr=single
  Constant1 : Constant  Value=0.5  OutDataTypeStr=single
  Product : Product  Inputs=5  OutDataTypeStr=single
  Product1 : Product  Inputs=4  OutDataTypeStr=single
  Product2 : Product  OutDataTypeStr=single
  distPhase2 : Outport
  wires:
  Add/1 -> distPhase2/1
  Product1/1 -> Add/1
  Product/1 -> Add/3
  Product2/1 -> Add/2
  subjVel/1 -> Product2/1
  Constant1/1 -> Product1/1
  subjAccelFilt/1 -> Product1/2
  Constant/1 -> Product/5
  Kjerk/1 -> Product/4
  tPhase2/1 -> Product/3, Product/2, Product/1, Product2/2, Product1/4, Product1/3

== system TosAeb/FcwAebJa_Step_sys/ALG_AEBSystem/ALG_AEBController/AEB_LatObjInfoCalcApp/LatCycObjCalc/LatCycCollisonAssessInfoCalc/LatCycCollisonAssessInfoCalc/DistBrkCondCalc/CycDistBrakePartCalc/DistPhase2Calc/tPhase2Calc ==
  note: t jerk_0 = （ -a ego /K jer k ） + sqrt （（ a ego /K jer k ） ²- 2* （ v ego / K jer k ））
  note: t jerk_amax = Max[0 ，（ a m ax - a ego ） /K jerk ] ；
  subjVel : Inport
  Kjerk : Inport  Port=2  OutDataTypeStr=single
  subjLongAccelFilt : Inport  Port=3  OutDataTypeStr=single
  accelMax : Inport  Port=4  OutDataTypeStr=single
  Add : Sum  Inputs=+-  OutDataTypeStr=single
  Add1 : Sum  Inputs=-+  OutDataTypeStr=single
  Add2 : Sum  Inputs=+-  OutDataTypeStr=single
  Constant : Constant  Value=0  OutDataTypeStr=single
  Divide : Product  Inputs=*/  OutDataTypeStr=single
  Divide1 : Product  Inputs=*/  OutDataTypeStr=single
  Divide2 : Product  Inputs=*/  OutDataTypeStr=single
  Gain : Gain  Gain=2  OutDataTypeStr=single
  Math Function : Math  Operator=square  SignedPower=on  OutDataTypeStr=single  SaturateOnIntegerOverflow=on
  MinMax : MinMax  Function=max  Inputs=2  OutDataTypeStr=single
  MinMax1 : MinMax  Inputs=2  OutDataTypeStr=single
  Relational Operator : RelationalOperator  Operator=<=  OutDataTypeStr=boolean  RndMeth=Simplest
  Sqrt : Sqrt  OutDataTypeStr=single  SaturateOnIntegerOverflow=on
  egoStopFlg : Outport
  tJerkWork : Outport  Port=2
  tPhase2 : Outport  Port=3
  wires:
  Relational Operator/1 -> egoStopFlg/1
  subjVel/1 -> Divide1/1
  Math Function/1 -> Add/1
  Add1/1 -> MinMax1/2, Relational Operator/1  "tEgoStop"
  Gain/1 -> Add/2
  Add/1 -> Sqrt/1
  Divide1/1 -> Gain/1
  MinMax1/1 -> tPhase2/1
  Divide/1 -> Math Function/1, Add1/1
  Constant/1 -> MinMax/2
  Divide2/1 -> MinMax/1
  accelMax/1 -> Add2/1
  Kjerk/1 -> Divide1/2, Divide/2, Divide2/2
  Sqrt/1 -> Add1/2
  subjLongAccelFilt/1 -> Divide/1, Add2/2
  MinMax/1 -> MinMax1/1, Relational Operator/2, tJerkWork/1  "tJerkWork"
  Add2/1 -> Divide2/1

== system TosAeb/FcwAebJa_Step_sys/ALG_AEBSystem/ALG_AEBController/AEB_LatObjInfoCalcApp/LatCycObjCalc/LatCycCollisonAssessInfoCalc/LatCycCollisonAssessInfoCalc/DistBrkCondCalc/CycDistBrakePartCalc/DistPhase3Calc/distPhase3Calc ==
  note: d3=（vego+ 0.5* aego*（t1 + t2）+ 0.5* Kjerk* t2²）*t3 + 0.5* amax* t3²；
  tPhase3 : Inport  OutDataTypeStr=single
  subjVel : Inport  Port=2
  subjLongAccelFilt : Inport  Port=3  OutDataTypeStr=single
  Kjerk : Inport  Port=4  OutDataTypeStr=single
  tPhase1 : Inport  Port=5  OutDataTypeStr=single
  tPhase2 : Inport  Port=6  OutDataTypeStr=single
  accelMax : Inport  Port=7  OutDataTypeStr=single
  Add : Sum  OutDataTypeStr=single
  Add1 : Sum  Inputs=+++  OutDataTypeStr=single
  Add2 : Sum  OutDataTypeStr=single
  Constant : Constant  Value=0.5  OutDataTypeStr=single
  Constant1 : Constant  Value=0.5  OutDataTypeStr=single
  Constant2 : Constant  Value=0.5  OutDataTypeStr=single
  Product : Product  Inputs=3  OutDataTypeStr=single
  Product1 : Product  Inputs=4  OutDataTypeStr=single
  Product2 : Product  OutDataTypeStr=single
  Product3 : Product  Inputs=4  OutDataTypeStr=single
  distPhase3 : Outport
  wires:
  Add2/1 -> distPhase3/1
  Product2/1 -> Add2/2
  Product3/1 -> Add2/1
  accelMax/1 -> Product3/2
  Constant2/1 -> Product3/1
  tPhase3/1 -> Product3/3, Product3/4, Product2/1
  Add1/1 -> Product2/2
  subjVel/1 -> Add1/1
  Product1/1 -> Add1/3
  Product/1 -> Add1/2
  Kjerk/1 -> Product1/3
  Constant1/1 -> Product1/4
  Constant/1 -> Product/1
  Add/1 -> Product/3
  subjLongAccelFilt/1 -> Product/2
  tPhase2/1 -> Add/2, Product1/1, Product1/2
  tPhase1/1 -> Add/1

== system TosAeb/FcwAebJa_Step_sys/ALG_AEBSystem/ALG_AEBController/AEB_LatObjInfoCalcApp/LatCycObjCalc/LatCycCollisonAssessInfoCalc/LatCycCollisonAssessInfoCalc/DistBrkCondCalc/CycDistBrakePartCalc/DistPhase3Calc/tPhase3Calc ==
  subjVel : Inport
  subjLongAccelFilt : Inport  Port=2  OutDataTypeStr=single
  tJerkWork : Inport  Port=3  OutDataTypeStr=single
  Kjerk : Inport  Port=4  OutDataTypeStr=single
  accelMax : Inport  Port=5  OutDataTypeStr=single
  velDelta : Inport  Port=6  OutDataTypeStr=single
  Action Port : ActionPort  ActionPortLabel=else
  Bus Creator : BusCreator  Inputs=6
  Bus Selector : BusSelector  OutputSignals=velDelta,subjLongAccelFilt,tJerkWork,Kjerk,accelMax
  Bus Selector1 : BusSelector  OutputSignals=subjVel,subjLongAccelFilt,tJerkWork,Kjerk,accelMax
  tEgoStopCalc : Subsystem
  tPhase3Calc : Subsystem
  tVelDeltaCalc : Subsystem
  tPhase3 : Outport
  wires:
  Bus Selector1/5 -> tEgoStopCalc/5(accelMax)  "<accelMax>"
  Bus Selector1/4 -> tEgoStopCalc/4(Kjerk)  "<Kjerk>"
  Bus Selector1/3 -> tEgoStopCalc/3(tJerkWork)  "<tJerkWork>"
  Bus Selector1/2 -> tEgoStopCalc/2(subjLongAccelFilt)  "<subjLongAccelFilt>"
  Bus Selector1/1 -> tEgoStopCalc/1(subjVel)  "<subjVel>"
  Bus Selector/5 -> tVelDeltaCalc/5(accelMax)  "<accelMax>"
  Bus Selector/1 -> tVelDeltaCalc/1(velDelta)  "<velDelta>"
  Bus Selector/4 -> tVelDeltaCalc/4(Kjerk)  "<Kjerk>"
  Bus Selector/2 -> tVelDeltaCalc/2(subjLongAccelFilt)  "<subjLongAccelFilt>"
  Bus Selector/3 -> tVelDeltaCalc/3(tJerkWork)  "<tJerkWork>"
  Bus Creator/1 -> Bus Selector/1, Bus Selector1/1
  velDelta/1 -> Bus Creator/6  "velDelta"
  accelMax/1 -> Bus Creator/5  "accelMax"
  Kjerk/1 -> Bus Creator/4  "Kjerk"
  tJerkWork/1 -> Bus Creator/3  "tJerkWork"
  subjLongAccelFilt/1 -> Bus Creator/2  "subjLongAccelFilt"
  subjVel/1 -> Bus Creator/1  "subjVel"
  tPhase3Calc/1(tPhase3) -> tPhase3/1
  tEgoStopCalc/1(tEgoStop) -> tPhase3Calc/2(tEgoStop)
  tVelDeltaCalc/1(tVelDelta) -> tPhase3Calc/1(tVelDelta)

== system TosAeb/FcwAebJa_Step_sys/ALG_AEBSystem/ALG_AEBController/AEB_LatObjInfoCalcApp/LatCycObjCalc/LatCycCollisonAssessInfoCalc/LatCycCollisonAssessInfoCalc/DistBrkCondCalc/CycDistBrakePartCalc/DistPhase3Calc/tPhase3Calc1 ==
  Action Port : ActionPort  ActionPortLabel=if(u1)
  Constant : Constant  Value=0  OutDataTypeStr=single
  tPhase3 : Outport
  wires:
  Constant/1 -> tPhase3/1

== system TosAeb/FcwAebJa_Step_sys/ALG_AEBSystem/ALG_AEBController/AEB_LatObjInfoCalcApp/LatCycObjCalc/LatCycCollisonAssessInfoCalc/LatCycCollisonAssessInfoCalc/DistBrkCondCalc/distBraking4FullBrakeCalc1/DistPhase2Calc/distPhase2Calc ==
  tWorkPhase2 : Inport  OutDataTypeStr=single
  subjVel : Inport  Port=2
  Kjerk : Inport  Port=3  OutDataTypeStr=single
  subjLongAccelCorr : Inport  Port=4  OutDataTypeStr=single
  Add2 : Sum  Inputs=+++  OutDataTypeStr=single
  Gain1 : Gain  Gain=0.5  OutDataTypeStr=single
  Gain3 : Gain  Gain=1/6  OutDataTypeStr=single
  Math Function1 : Math  Operator=square  SignedPower=on  OutDataTypeStr=single  SaturateOnIntegerOverflow=on
  Product1 : Product  OutDataTypeStr=single
  Product2 : Product  Inputs=4  OutDataTypeStr=single
  Product6 : Product  OutDataTypeStr=single
  distPhase2 : Outport
  wires:
  subjLongAccelCorr/1 -> Product6/2
  Gain3/1 -> Add2/1
  subjVel/1 -> Product1/2
  Add2/1 -> distPhase2/1
  Product6/1 -> Gain1/1
  Product2/1 -> Gain3/1
  Product1/1 -> Add2/3
  Math Function1/1 -> Product6/1
  tWorkPhase2/1 -> Product2/2, Product2/3, Product2/4, Math Function1/1, Product1/1
  Kjerk/1 -> Product2/1
  Gain1/1 -> Add2/2

== system TosAeb/FcwAebJa_Step_sys/ALG_AEBSystem/ALG_AEBController/AEB_LatObjInfoCalcApp/LatCycObjCalc/LatCycCollisonAssessInfoCalc/LatCycCollisonAssessInfoCalc/DistBrkCondCalc/distBraking4FullBrakeCalc1/DistPhase2Calc/tWorkPhase2Calc ==
  note: 缺少系数2
  accelMax : Inport  OutDataTypeStr=single
  accelWorkPhase1 : Inport  Port=2  OutDataTypeStr=single
  Kjerk : Inport  Port=3  OutDataTypeStr=single
  subjVel : Inport  Port=4
  Add : Sum  Inputs=+-  OutDataTypeStr=single
  Add1 : Sum  OutDataTypeStr=single
  Divide : Product  Inputs=*/  OutDataTypeStr=single
  Divide1 : Product  Inputs=*/  OutDataTypeStr=single
  Divide2 : Product  Inputs=*/  OutDataTypeStr=single
  Gain : Gain  Gain=-1  OutDataTypeStr=single
  Gain1 : Gain  Gain=2  OutDataTypeStr=single
  Math Function : Math  Operator=square  SignedPower=on  OutDataTypeStr=single  SaturateOnIntegerOverflow=on
  MinMax : MinMax  Inputs=2  OutDataTypeStr=single
  Relational Operator1 : RelationalOperator  Operator=<  OutDataTypeStr=boolean  RndMeth=Simplest
  Sqrt : Sqrt  OutDataTypeStr=single  SaturateOnIntegerOverflow=on
  Subtract : Sum  Inputs=+-  OutDataTypeStr=single
  Phase2EgoStopFlg : Outport
  tWorkPhase2 : Outport  Port=2
  wires:
  Relational Operator1/1 -> Phase2EgoStopFlg/1
  MinMax/1 -> tWorkPhase2/1
  Sqrt/1 -> Add1/2
  Gain/1 -> Add1/1
  Add/1 -> Sqrt/1
  Subtract/1 -> Divide2/1
  accelMax/1 -> Subtract/1
  Add1/1 -> Relational Operator1/1, MinMax/2
  accelWorkPhase1/1 -> Divide/1, Subtract/2
  Divide1/1 -> Gain1/1
  Divide/1 -> Gain/1, Math Function/1
  Math Function/1 -> Add/1
  subjVel/1 -> Divide1/1
  [Kjerk] -> Divide2/2
  Kjerk/1 -> [Kjerk], Divide1/2, Divide/2
  Divide2/1 -> Relational Operator1/2, MinMax/1
  Gain1/1 -> Add/2

== system TosAeb/FcwAebJa_Step_sys/ALG_AEBSystem/ALG_AEBController/AEB_LatObjInfoCalcApp/LatCycObjCalc/LatCycCollisonAssessInfoCalc/LatCycCollisonAssessInfoCalc/DistBrkCondCalc/distBraking4FullBrakeCalc1/distPhase3Calc/EgoStopped ==
  Action Port : ActionPort
  Constant : Constant  Value=0  OutDataTypeStr=single
  distPhase3 : Outport
  wires:
  Constant/1 -> distPhase3/1

== system TosAeb/FcwAebJa_Step_sys/ALG_AEBSystem/ALG_AEBController/AEB_LatObjInfoCalcApp/LatCycObjCalc/LatCycCollisonAssessInfoCalc/LatCycCollisonAssessInfoCalc/DistBrkCondCalc/distBraking4FullBrakeCalc1/distPhase3Calc/distPhase3Calc ==
  Kjerk : Inport  OutDataTypeStr=single
  tWorkPhase2 : Inport  Port=2  OutDataTypeStr=single
  accelWorkPhase1 : Inport  Port=3  OutDataTypeStr=single
  subjLongAccelCorr : Inport  Port=4  OutDataTypeStr=single
  velMaxReduce : Inport  Port=5  OutDataTypeStr=single
  accelMax : Inport  Port=6  OutDataTypeStr=single
  tWorkPhase1 : Inport  Port=7  OutDataTypeStr=single
  Action Port : ActionPort
  distPhase3Calc : Subsystem
  subjVelPhase2Calc : Subsystem
  tWorktimePhase3 : Subsystem
  distPhase3 : Outport
  wires:
  tWorkPhase1/1 -> subjVelPhase2Calc/5(tWorkPhase1)
  subjLongAccelCorr/1 -> subjVelPhase2Calc/4(subjLongAccelCorr)
  accelWorkPhase1/1 -> subjVelPhase2Calc/3(accelWorkPhase1)
  tWorkPhase2/1 -> subjVelPhase2Calc/2(tWorkPhase2)
  Kjerk/1 -> subjVelPhase2Calc/1(Kjerk)
  distPhase3Calc/1(distPhase3) -> distPhase3/1
  subjVelPhase2Calc/2(subjVelPhase2) -> distPhase3Calc/3(subjVelPhase2), tWorktimePhase3/4(subjVelPhase2)
  tWorktimePhase3/1(tWorkPhase3) -> distPhase3Calc/2(tWorkPhase3)
  [accelMax] -> distPhase3Calc/1(accelMax)
  velMaxReduce/1 -> tWorktimePhase3/1(velMaxReduce)
  accelMax/1 -> [accelMax], tWorktimePhase3/2(accelMax)
  subjVelPhase2Calc/1(subjVelReducePhase2) -> tWorktimePhase3/3(subjVelReducePhase2)

== system TosAeb/FcwAebJa_Step_sys/ALG_AEBSystem/ALG_AEBController/AEB_LatObjInfoCalcApp/LatCycObjCalc/LatCycCollisonAssessInfoCalc/LatCycCollisonAssessInfoCalc/DistBrkCondCalc/distBraking4PartBrakeCalc1/DistPhase2Calc/distPhase2Calc ==
  tWorkPhase2 : Inport  OutDataTypeStr=single
  subjVel : Inport  Port=2
  Kjerk : Inport  Port=3  OutDataTypeStr=single
  subjLongAccelCorr : Inport  Port=4  OutDataTypeStr=single
  Add2 : Sum  Inputs=+++  OutDataTypeStr=single
  Gain1 : Gain  Gain=0.5  OutDataTypeStr=single
  Gain3 : Gain  Gain=1/6  OutDataTypeStr=single
  Math Function1 : Math  Operator=square  SignedPower=on  OutDataTypeStr=single  SaturateOnIntegerOverflow=on
  Product1 : Product  OutDataTypeStr=single
  Product2 : Product  Inputs=4  OutDataTypeStr=single
  Product6 : Product  OutDataTypeStr=single
  distPhase2 : Outport
  wires:
  Gain1/1 -> Add2/2
  Kjerk/1 -> Product2/1
  tWorkPhase2/1 -> Product1/1, Math Function1/1, Product2/4, Product2/3, Product2/2
  Math Function1/1 -> Product6/1
  Product1/1 -> Add2/3
  Product2/1 -> Gain3/1
  Product6/1 -> Gain1/1
  Add2/1 -> distPhase2/1
  subjVel/1 -> Product1/2
  Gain3/1 -> Add2/1
  subjLongAccelCorr/1 -> Product6/2

== system TosAeb/FcwAebJa_Step_sys/ALG_AEBSystem/ALG_AEBController/AEB_LatObjInfoCalcApp/LatCycObjCalc/LatCycCollisonAssessInfoCalc/LatCycCollisonAssessInfoCalc/DistBrkCondCalc/distBraking4PartBrakeCalc1/DistPhase2Calc/tWorkPhase2Calc ==
  note: 缺少系数2
  accelMax : Inport  OutDataTypeStr=single
  accelWorkPhase1 : Inport  Port=2  OutDataTypeStr=single
  Kjerk : Inport  Port=3  OutDataTypeStr=single
  subjVel : Inport  Port=4
  Add : Sum  Inputs=+-  OutDataTypeStr=single
  Add1 : Sum  OutDataTypeStr=single
  Divide : Product  Inputs=*/  OutDataTypeStr=single
  Divide1 : Product  Inputs=*/  OutDataTypeStr=single
  Divide2 : Product  Inputs=*/  OutDataTypeStr=single
  Gain : Gain  Gain=-1  OutDataTypeStr=single
  Gain1 : Gain  Gain=2  OutDataTypeStr=single
  Math Function : Math  Operator=square  SignedPower=on  OutDataTypeStr=single  SaturateOnIntegerOverflow=on
  MinMax : MinMax  Inputs=2  OutDataTypeStr=single
  Relational Operator1 : RelationalOperator  Operator=<  OutDataTypeStr=boolean  RndMeth=Simplest
  Sqrt : Sqrt  OutDataTypeStr=single  SaturateOnIntegerOverflow=on
  Subtract : Sum  Inputs=+-  OutDataTypeStr=single
  Phase2EgoStopFlg : Outport
  tWorkPhase2 : Outport  Port=2
  wires:
  Gain1/1 -> Add/2
  Divide2/1 -> MinMax/1, Relational Operator1/2
  Kjerk/1 -> Divide/2, Divide1/2, [Kjerk]
  [Kjerk] -> Divide2/2
  subjVel/1 -> Divide1/1
  Math Function/1 -> Add/1
  Divide/1 -> Math Function/1, Gain/1
  Divide1/1 -> Gain1/1
  accelWorkPhase1/1 -> Subtract/2, Divide/1
  Add1/1 -> MinMax/2, Relational Operator1/1
  accelMax/1 -> Subtract/1
  Subtract/1 -> Divide2/1
  Add/1 -> Sqrt/1
  Gain/1 -> Add1/1
  Sqrt/1 -> Add1/2
  MinMax/1 -> tWorkPhase2/1
  Relational Operator1/1 -> Phase2EgoStopFlg/1

== system TosAeb/FcwAebJa_Step_sys/ALG_AEBSystem/ALG_AEBController/AEB_LatObjInfoCalcApp/LatCycObjCalc/LatCycCollisonAssessInfoCalc/LatCycCollisonAssessInfoCalc/DistBrkCondCalc/distBraking4PartBrakeCalc1/distPhase3Calc/EgoStopped ==
  Action Port : ActionPort
  Constant : Constant  Value=0  OutDataTypeStr=single
  distPhase3 : Outport
  wires:
  Constant/1 -> distPhase3/1

== system TosAeb/FcwAebJa_Step_sys/ALG_AEBSystem/ALG_AEBController/AEB_LatObjInfoCalcApp/LatCycObjCalc/LatCycCollisonAssessInfoCalc/LatCycCollisonAssessInfoCalc/DistBrkCondCalc/distBraking4PartBrakeCalc1/distPhase3Calc/distPhase3Calc ==
  Kjerk : Inport  OutDataTypeStr=single
  tWorkPhase2 : Inport  Port=2  OutDataTypeStr=single
  accelWorkPhase1 : Inport  Port=3  OutDataTypeStr=single
  subjLongAccelCorr : Inport  Port=4  OutDataTypeStr=single
  velMaxReduce : Inport  Port=5  OutDataTypeStr=single
  accelMax : Inport  Port=6  OutDataTypeStr=single
  tWorkPhase1 : Inport  Port=7  OutDataTypeStr=single
  Action Port : ActionPort
  distPhase3Calc : Subsystem
  subjVelPhase2Calc : Subsystem
  tWorktimePhase3 : Subsystem
  distPhase3 : Outport
  wires:
  subjVelPhase2Calc/1(subjVelReducePhase2) -> tWorktimePhase3/3(subjVelReducePhase2)
  accelMax/1 -> tWorktimePhase3/2(accelMax), [accelMax]
  velMaxReduce/1 -> tWorktimePhase3/1(velMaxReduce)
  [accelMax] -> distPhase3Calc/1(accelMax)
  tWorktimePhase3/1(tWorkPhase3) -> distPhase3Calc/2(tWorkPhase3)
  subjVelPhase2Calc/2(subjVelPhase2) -> tWorktimePhase3/4(subjVelPhase2), distPhase3Calc/3(subjVelPhase2)
  distPhase3Calc/1(distPhase3) -> distPhase3/1
  Kjerk/1 -> subjVelPhase2Calc/1(Kjerk)
  tWorkPhase2/1 -> subjVelPhase2Calc/2(tWorkPhase2)
  accelWorkPhase1/1 -> subjVelPhase2Calc/3(accelWorkPhase1)
  subjLongAccelCorr/1 -> subjVelPhase2Calc/4(subjLongAccelCorr)
  tWorkPhase1/1 -> subjVelPhase2Calc/5(tWorkPhase1)
